simulink model "large_model"  (Simulink 8.0)
settings: Solver=ode45  StartTime=0.0  StopTime=10.0  RelTol=1e-3
(part 7 of 21)

== system large_model/Integrated_GPM1_4/End_System ==
  From_GPM : Inport
  From_Switch_ChA : Inport  Port=2
  From_Switch_ChB : Inport  Port=3
  PWR : Inport  Port=4
  Bus Creator : BusCreator  Inputs=2
  ES Hardware : Subsystem
  To_GPM : Outport
  To_Switch_ChA : Outport  Port=2
  To_Switch_ChB : Outport  Port=3
  wires:
  From_GPM/1 -> ES Hardware/1(In1)
  Bus Creator/1 -> ES Hardware/2(In2)
  PWR/1 -> ES Hardware/3(PWR)
  ES Hardware/2(Out2) -> To_GPM/1
  ES Hardware/1(Out1) -> To_Switch_ChB/1, To_Switch_ChA/1
  From_Switch_ChB/1 -> Bus Creator/2
  From_Switch_ChA/1 -> Bus Creator/1
